simulink model "AcasXuClosedLoop_all_2017b"  (Simulink 9.0)
settings: Solver=VariableStepAuto  StartTime=0.0  StopTime=20  RelTol=1e-3
libraries: neural x14040, simulink x2
(part 15 of 534)

== system AcasXuClosedLoop_all_2017b/networks/Networks_m_3/AcasXu_3_3/Layer 3 ==
  "a{2} " : Inport  PortDimensions=50
      mask Display: e = 0.06;c1 = [120 230 180]/255;c2 = ([120 230 180]+0)/(256+128);c3 = ([120 230 180]+128)/(256+128);patch([0 1 1 0],[0 0 1 1],c1);patch([0 1 1 1-e 1-e 0+e],[0 0 1 1-e 0+e 0+e],c2);patch([0 0 1 1-e 0+e 0+e],[0 1 1 1-e 1-e 0+e],c3);plot([0 1 1 0 0],[0 0 1 1 0]);
  Delays 1 : Subsystem
      mask Display: e = 0.06;c1 = [120 150 230]/255;c2 = ([120 150 230]+0)/383;c3 = ([120 150 230]+128)/383;patch([0 1 1 0],[0 0 1 1],c1);patch([0 1 1 1-e 1-e 0+e],[0 0 1 1-e 0+e 0+e],c2);patch([0 0 1 1-e 0+e 0+e],[0 1 1 1-e 1-e 0+e],c3);plot([0 1 1 0 0],[0 0 1 1 0]);disp('0');
  "LW{3,2}" : Subsystem
      mask Display: e = 0.06;c1 = [120 150 230]/255;c2 = ([120 150 230]+0)/383;c3 = ([120 150 230]+128)/383;patch([0 1 1 0],[0 0 1 1],c1);patch([0 1 1 1-e 1-e 0+e],[0 0 1 1-e 0+e 0+e],c2);patch([0 0 1 1-e 0+e 0+e],[0 1 1 1-e 1-e 0+e],c3);plot([0 1 1 0 0],[0 0 1 1 0]);disp('W');
  "b{3}" : Constant  Value=[1.457030000000000047322146201622672379016876220703125;0.269340999999999997083222069704788736999034881591796875;-0.0234063000000000014100276501949338125996291637420654296875;-1.55862999999999995992538970313034951686859130859375;-0.578284000000000020236257114447653293609619140625;-0.79902899999999998925659383530728518962860107421875;-1.995579999999999909476855464163236320018768310546875;-2.194290000000000073754335971898399293422698974609375;-0.2367740000000000122337695529495249502360820770263671875;-0.71987100000000003863220854327664710581302642822265625;-0.10746999999999999608757406122094835154712200164794921875;-1.548810000000000020037305148434825241565704345703125;-1.0187399999999999788968807479250244796276092529296875;-0.328844999999999998419042412933777086436748504638671875;-1.9179999999999999271693695845897309482097625732421875;-1.38178000000000000824229573481716215610504150390625;-1.721340000000000092228447101661004126071929931640625;-1.3627000000000000223820961764431558549404144287109375;-0.007110700000000000180200299126909158076159656047821044921875;-0.8580069999999999641460135535453446209430694580078125;0.2330230000000000079030115784917143173515796661376953125;0.6196960000000000245989895120146684348583221435546875;0.478331000000000006178169087434071116149425506591796875;-1.4873700000000000809308176030754111707210540771484375;0.499203000000000007840839089112705551087856292724609375;0.1549939999999999928892435718807973898947238922119140625;-0.72241400000000000058975047068088315427303314208984375;-0.9493819999999999481588019989430904388427734375;0.0788308000000000064222405171676655299961566925048828125;0.66645100000000001561062390464940108358860015869140625;0.2498390000000000055191407000165781937539577484130859375;-0.0307758999999999983965270899943789117969572544097900390625;0.60483399999999998275512780310236848890781402587890625;-1.92368000000000005655920176650397479534149169921875;-0.333158000000000009688250202088966034352779388427734375;-0.58032399999999995099386751462589018046855926513671875;-0.422524999999999983923970603427733294665813446044921875;-1.3587100000000000843414227347238920629024505615234375;-0.50581399999999998584598870365880429744720458984375;-1.099110000000000031405988920596428215503692626953125;0.245719999999999993978150314433150924742221832275390625;-1.059979999999999922266624707845039665699005126953125;0.105677999999999994162891425730776973068714141845703125;-0.512558999999999986840748533722944557666778564453125;-0.53886000000000000564881474929279647767543792724609375;0.335861000000000020637713760152109898626804351806640625;-2.210109999999999796926886119763366878032684326171875;1.146900000000000030553337637684307992458343505859375;-2.13619000000000003325340003357268869876861572265625;-1.1020000000000000905941988094127736985683441162109375]
      mask Display: e = 0.06;c1 = [120 150 230]/255;c2 = ([120 150 230]+0)/383;c3 = ([120 150 230]+128)/383;patch([0 1 1 0],[0 0 1 1],c1);patch([0 1 1 1-e 1-e 0+e],[0 0 1 1-e 0+e 0+e],c2);patch([0 0 1 1-e 0+e 0+e],[0 1 1 1-e 1-e 0+e],c3);plot([0 1 1 0 0],[0 0 1 1 0]);disp('b');
  netsum : Sum  Inputs=2  SaturateOnIntegerOverflow=on  InputSameDT=on  OutDataTypeStr=Inherit: Same as first input
      mask Display: e = 0.06;c1 = [120 150 230]/255;c2 = ([120 150 230]+0)/383;c3 = ([120 150 230]+128)/383;patch([0 1 1 0],[0 0 1 1],c1);patch([0 1 1 1-e 1-e 0+e],[0 0 1 1-e 0+e 0+e],c2);patch([0 0 1 1-e 0+e 0+e],[0 1 1 1-e 1-e 0+e],c3);plot([0 1 1 0 0],[0 0 1 1 0]);disp('+');
  poslin : neural/Transfer Functions/poslin
      settings: SourceProductName=Deep Learning Toolbox  SourceProductBaseCode=NN
  "a{3}" : Outport  InitialOutput=[0;0;0;0;0;0;0;0;0;0;0;0;0;0;0;0;0;0;0;0;0;0;0;0;0;0;0;0;0;0;0;0;0;0;0;0;0;0;0;0;0;0;0;0;0;0;0;0;0;0]
      mask Display: e = 0.06;c1 = [120 230 180]/255;c2 = ([120 230 180]+0)/(256+128);c3 = ([120 230 180]+128)/(256+128);patch([0 1 1 0],[0 0 1 1],c1);patch([0 1 1 1-e 1-e 0+e],[0 0 1 1-e 0+e 0+e],c2);patch([0 0 1 1-e 0+e 0+e],[0 1 1 1-e 1-e 0+e],c3);plot([0 1 1 0 0],[0 0 1 1 0]);
  wires:
  poslin/1 -> "a{3}"/1
  netsum/1 -> poslin/1
  "b{3}"/1 -> netsum/2
  "LW{3,2}"/1("lz{3,2}") -> netsum/1
  Delays 1/1("pd{3,2}") -> "LW{3,2}"/1("ad{3,2}")
  "a{2} "/1 -> Delays 1/1("p{3}")

== system AcasXuClosedLoop_all_2017b/networks/Networks_m_3/AcasXu_3_3/Layer 4 ==
  "a{3} " : Inport  PortDimensions=50
      mask Display: e = 0.06;c1 = [120 230 180]/255;c2 = ([120 230 180]+0)/(256+128);c3 = ([120 230 180]+128)/(256+128);patch([0 1 1 0],[0 0 1 1],c1);patch([0 1 1 1-e 1-e 0+e],[0 0 1 1-e 0+e 0+e],c2);patch([0 0 1 1-e 0+e 0+e],[0 1 1 1-e 1-e 0+e],c3);plot([0 1 1 0 0],[0 0 1 1 0]);
  Delays 1 : Subsystem
      mask Display: e = 0.06;c1 = [120 150 230]/255;c2 = ([120 150 230]+0)/383;c3 = ([120 150 230]+128)/383;patch([0 1 1 0],[0 0 1 1],c1);patch([0 1 1 1-e 1-e 0+e],[0 0 1 1-e 0+e 0+e],c2);patch([0 0 1 1-e 0+e 0+e],[0 1 1 1-e 1-e 0+e],c3);plot([0 1 1 0 0],[0 0 1 1 0]);disp('0');
  "LW{4,3}" : Subsystem
      mask Display: e = 0.06;c1 = [120 150 230]/255;c2 = ([120 150 230]+0)/383;c3 = ([120 150 230]+128)/383;patch([0 1 1 0],[0 0 1 1],c1);patch([0 1 1 1-e 1-e 0+e],[0 0 1 1-e 0+e 0+e],c2);patch([0 0 1 1-e 0+e 0+e],[0 1 1 1-e 1-e 0+e],c3);plot([0 1 1 0 0],[0 0 1 1 0]);disp('W');
  "b{4}" : Constant  Value=[0.71493099999999998317434801720082759857177734375;0.02434619999999999839790376654491410590708255767822265625;-1.1054600000000001092104184863273985683917999267578125;0.0755138999999999949164219970043632201850414276123046875;0.1088739999999999985558218895675963722169399261474609375;0.34726800000000002111022467943257652223110198974609375;0.246087000000000000188293824976426549255847930908203125;0.0086718999999999997474464663582693901844322681427001953125;0.94580399999999997806554574708570726215839385986328125;-1.1706499999999999683808482586755417287349700927734375;-0.5646409999999999484998625121079385280609130859375;-0.779117999999999977234210746246390044689178466796875;-0.4107439999999999979962694851565174758434295654296875;0.710671000000000052665427574538625776767730712890625;0.8943090000000000205915284823277033865451812744140625;-0.40828900000000001302424834648263640701770782470703125;-2.18846000000000007190692485892213881015777587890625;1.024010000000000086828322309884242713451385498046875;1.003929999999999989057641869294457137584686279296875;0.403766000000000013780976360067143104970455169677734375;1.5085900000000000975575176198617555201053619384765625;-1.2966599999999999237587644529412500560283660888671875;0.547646999999999994912514011957682669162750244140625;-2.286960000000000103881347968126647174358367919921875;-0.01220700000000000083610895984520539059303700923919677734375;-0.3039169999999999927098315311013720929622650146484375;1.2535600000000000076028072726330719888210296630859375;-0.562553999999999998493649400188587605953216552734375;-0.12156999999999999750865953274114872328937053680419921875;0.69034099999999998242827814465272240340709686279296875;-0.10991299999999999681410400853565079160034656524658203125;0.422111999999999987220888897354598157107830047607421875;-0.457382000000000010775380587801919318735599517822265625;-0.274575999999999986744825264395331032574176788330078125;0.3055309999999999970299313645227812230587005615234375;-1.1061199999999999921129756330628879368305206298828125;0.905712999999999990308197084232233464717864990234375;0.27538099999999998690469737994135357439517974853515625;-0.2375750000000000083932860661661834456026554107666015625;0.79457199999999994499688682481064461171627044677734375;-1.9045900000000000051869619710487313568592071533203125;0.16324600000000000221689333557151257991790771484375;0.11122700000000000641886543917280505411326885223388671875;1.0326299999999999368327507909270934760570526123046875;1.5072700000000001097077984013594686985015869140625;0.32911400000000001764277612892328761518001556396484375;1.3003899999999999348432311307988129556179046630859375;0.25018499999999999072741729833069257438182830810546875;1.8247400000000000286348722511320374906063079833984375;0.362935999999999980847320557586499489843845367431640625]
      mask Display: e = 0.06;c1 = [120 150 230]/255;c2 = ([120 150 230]+0)/383;c3 = ([120 150 230]+128)/383;patch([0 1 1 0],[0 0 1 1],c1);patch([0 1 1 1-e 1-e 0+e],[0 0 1 1-e 0+e 0+e],c2);patch([0 0 1 1-e 0+e 0+e],[0 1 1 1-e 1-e 0+e],c3);plot([0 1 1 0 0],[0 0 1 1 0]);disp('b');
  netsum : Sum  Inputs=2  SaturateOnIntegerOverflow=on  InputSameDT=on  OutDataTypeStr=Inherit: Same as first input
      mask Display: e = 0.06;c1 = [120 150 230]/255;c2 = ([120 150 230]+0)/383;c3 = ([120 150 230]+128)/383;patch([0 1 1 0],[0 0 1 1],c1);patch([0 1 1 1-e 1-e 0+e],[0 0 1 1-e 0+e 0+e],c2);patch([0 0 1 1-e 0+e 0+e],[0 1 1 1-e 1-e 0+e],c3);plot([0 1 1 0 0],[0 0 1 1 0]);disp('+');
  poslin : neural/Transfer Functions/poslin
      settings: SourceProductName=Deep Learning Toolbox  SourceProductBaseCode=NN
  "a{4}" : Outport  InitialOutput=[0;0;0;0;0;0;0;0;0;0;0;0;0;0;0;0;0;0;0;0;0;0;0;0;0;0;0;0;0;0;0;0;0;0;0;0;0;0;0;0;0;0;0;0;0;0;0;0;0;0]
      mask Display: e = 0.06;c1 = [120 230 180]/255;c2 = ([120 230 180]+0)/(256+128);c3 = ([120 230 180]+128)/(256+128);patch([0 1 1 0],[0 0 1 1],c1);patch([0 1 1 1-e 1-e 0+e],[0 0 1 1-e 0+e 0+e],c2);patch([0 0 1 1-e 0+e 0+e],[0 1 1 1-e 1-e 0+e],c3);plot([0 1 1 0 0],[0 0 1 1 0]);
  wires:
  poslin/1 -> "a{4}"/1
  netsum/1 -> poslin/1
  "b{4}"/1 -> netsum/2
  "LW{4,3}"/1("lz{4,3}") -> netsum/1
  Delays 1/1("pd{4,3}") -> "LW{4,3}"/1("ad{4,3}")
  "a{3} "/1 -> Delays 1/1("p{4}")

== system AcasXuClosedLoop_all_2017b/networks/Networks_m_3/AcasXu_3_3/Layer 5 ==
  "a{4} " : Inport  PortDimensions=50
      mask Display: e = 0.06;c1 = [120 230 180]/255;c2 = ([120 230 180]+0)/(256+128);c3 = ([120 230 180]+128)/(256+128);patch([0 1 1 0],[0 0 1 1],c1);patch([0 1 1 1-e 1-e 0+e],[0 0 1 1-e 0+e 0+e],c2);patch([0 0 1 1-e 0+e 0+e],[0 1 1 1-e 1-e 0+e],c3);plot([0 1 1 0 0],[0 0 1 1 0]);
  Delays 1 : Subsystem
      mask Display: e = 0.06;c1 = [120 150 230]/255;c2 = ([120 150 230]+0)/383;c3 = ([120 150 230]+128)/383;patch([0 1 1 0],[0 0 1 1],c1);patch([0 1 1 1-e 1-e 0+e],[0 0 1 1-e 0+e 0+e],c2);patch([0 0 1 1-e 0+e 0+e],[0 1 1 1-e 1-e 0+e],c3);plot([0 1 1 0 0],[0 0 1 1 0]);disp('0');
  "LW{5,4}" : Subsystem
      mask Display: e = 0.06;c1 = [120 150 230]/255;c2 = ([120 150 230]+0)/383;c3 = ([120 150 230]+128)/383;patch([0 1 1 0],[0 0 1 1],c1);patch([0 1 1 1-e 1-e 0+e],[0 0 1 1-e 0+e 0+e],c2);patch([0 0 1 1-e 0+e 0+e],[0 1 1 1-e 1-e 0+e],c3);plot([0 1 1 0 0],[0 0 1 1 0]);disp('W');
  "b{5}" : Constant  Value=[0.1411189999999999944435558063560165464878082275390625;-0.08221900000000000041655567883935873396694660186767578125;0.47773100000000001674749228186556138098239898681640625;1.2366800000000000014921397450962103903293609619140625;0.68673700000000004184386170891229994595050811767578125;-2.747520000000000184314785656169988214969635009765625;-2.62267999999999990023979989928193390369415283203125;-0.44178499999999998326671857284964062273502349853515625;0.50526800000000005042011252953670918941497802734375;0.295632999999999979134912564404658041894435882568359375;0.70687500000000003108624468950438313186168670654296875;1.251349999999999962341235004714690148830413818359375;0.2476069999999999937667638505445211194455623626708984375;-0.032298000000000000320188320301895146258175373077392578125;-0.255309000000000008157030606525950133800506591796875;-0.03110380000000000089155349769498570822179317474365234375;-0.093037800000000003830535888482700102031230926513671875;-0.0255745999999999994167776407039127661846578121185302734375;0.01323709999999999990805132910054453532211482524871826171875;-1.55783000000000004803268893738277256488800048828125;1.490650000000000030553337637684307992458343505859375;1.6610400000000000719779791324981488287448883056640625;0.34136100000000002552269506850279867649078369140625;-0.47167300000000000892441676114685833454132080078125;0.98957700000000003992539632236002944409847259521484375;-0.049506899999999999517630300260861986316740512847900390625;1.60648999999999997356781022972427308559417724609375;0.10922800000000000564259750035489560104906558990478515625;1.590100000000000068922645368729718029499053955078125;-1.5284999999999999698019337301957421004772186279296875;-1.85986999999999991217691786005161702632904052734375;0.37357899999999999440802866956801153719425201416015625;0.48918499999999998095745468162931501865386962890625;1.9168499999999999428013097713119350373744964599609375;1.475379999999999913740111878723837435245513916015625;-0.1451780000000000014903633882568101398646831512451171875;-1.4023399999999999199218336798367090523242950439453125;-0.280540999999999984826359877843060530722141265869140625;1.2049099999999999255351212923415005207061767578125;0.61131400000000002403766075076418928802013397216796875;-1.645399999999999973709918776876293122768402099609375;-0.88296600000000002861355596905923448503017425537109375;0.038886499999999997456701095188691397197544574737548828125;-0.315809999999999979625187052079127170145511627197265625;-1.0611399999999999721467247582040727138519287109375;0.870122999999999979792164594982750713825225830078125;-0.04864000000000000267785793539587757550179958343505859375;0.6608870000000000022311041902867145836353302001953125;-0.27579399999999998360777908601448871195316314697265625;-0.2108919999999999961293184469468542374670505523681640625]
      mask Display: e = 0.06;c1 = [120 150 230]/255;c2 = ([120 150 230]+0)/383;c3 = ([120 150 230]+128)/383;patch([0 1 1 0],[0 0 1 1],c1);patch([0 1 1 1-e 1-e 0+e],[0 0 1 1-e 0+e 0+e],c2);patch([0 0 1 1-e 0+e 0+e],[0 1 1 1-e 1-e 0+e],c3);plot([0 1 1 0 0],[0 0 1 1 0]);disp('b');
  netsum : Sum  Inputs=2  SaturateOnIntegerOverflow=on  InputSameDT=on  OutDataTypeStr=Inherit: Same as first input
      mask Display: e = 0.06;c1 = [120 150 230]/255;c2 = ([120 150 230]+0)/383;c3 = ([120 150 230]+128)/383;patch([0 1 1 0],[0 0 1 1],c1);patch([0 1 1 1-e 1-e 0+e],[0 0 1 1-e 0+e 0+e],c2);patch([0 0 1 1-e 0+e 0+e],[0 1 1 1-e 1-e 0+e],c3);plot([0 1 1 0 0],[0 0 1 1 0]);disp('+');
  poslin : neural/Transfer Functions/poslin
      settings: SourceProductName=Deep Learning Toolbox  SourceProductBaseCode=NN
  "a{5}" : Outport  InitialOutput=[0;0;0;0;0;0;0;0;0;0;0;0;0;0;0;0;0;0;0;0;0;0;0;0;0;0;0;0;0;0;0;0;0;0;0;0;0;0;0;0;0;0;0;0;0;0;0;0;0;0]
      mask Display: e = 0.06;c1 = [120 230 180]/255;c2 = ([120 230 180]+0)/(256+128);c3 = ([120 230 180]+128)/(256+128);patch([0 1 1 0],[0 0 1 1],c1);patch([0 1 1 1-e 1-e 0+e],[0 0 1 1-e 0+e 0+e],c2);patch([0 0 1 1-e 0+e 0+e],[0 1 1 1-e 1-e 0+e],c3);plot([0 1 1 0 0],[0 0 1 1 0]);
  wires:
  poslin/1 -> "a{5}"/1
  netsum/1 -> poslin/1
  "b{5}"/1 -> netsum/2
  "LW{5,4}"/1("lz{5,4}") -> netsum/1
  Delays 1/1("pd{5,4}") -> "LW{5,4}"/1("ad{5,4}")
  "a{4} "/1 -> Delays 1/1("p{5}")

== system AcasXuClosedLoop_all_2017b/networks/Networks_m_3/AcasXu_3_3/Layer 6 ==
  "a{5} " : Inport  PortDimensions=50
      mask Display: e = 0.06;c1 = [120 230 180]/255;c2 = ([120 230 180]+0)/(256+128);c3 = ([120 230 180]+128)/(256+128);patch([0 1 1 0],[0 0 1 1],c1);patch([0 1 1 1-e 1-e 0+e],[0 0 1 1-e 0+e 0+e],c2);patch([0 0 1 1-e 0+e 0+e],[0 1 1 1-e 1-e 0+e],c3);plot([0 1 1 0 0],[0 0 1 1 0]);
  Delays 1 : Subsystem
      mask Display: e = 0.06;c1 = [120 150 230]/255;c2 = ([120 150 230]+0)/383;c3 = ([120 150 230]+128)/383;patch([0 1 1 0],[0 0 1 1],c1);patch([0 1 1 1-e 1-e 0+e],[0 0 1 1-e 0+e 0+e],c2);patch([0 0 1 1-e 0+e 0+e],[0 1 1 1-e 1-e 0+e],c3);plot([0 1 1 0 0],[0 0 1 1 0]);disp('0');
  "LW{6,5}" : Subsystem
      mask Display: e = 0.06;c1 = [120 150 230]/255;c2 = ([120 150 230]+0)/383;c3 = ([120 150 230]+128)/383;patch([0 1 1 0],[0 0 1 1],c1);patch([0 1 1 1-e 1-e 0+e],[0 0 1 1-e 0+e 0+e],c2);patch([0 0 1 1-e 0+e 0+e],[0 1 1 1-e 1-e 0+e],c3);plot([0 1 1 0 0],[0 0 1 1 0]);disp('W');
  "b{6}" : Constant  Value=[0.0584027000000000018342660723646986298263072967529296875;-0.00940746999999999937436268027113328571431338787078857421875;-2.12145999999999990137666827649809420108795166015625;0.050215900000000000813482614603344700299203395843505859375;0.252966999999999997417177155512035824358463287353515625;0.460220000000000017958967646336532197892665863037109375;-0.09918480000000000351700890632855589501559734344482421875;-0.39645399999999997309174659676500596106052398681640625;-0.29279800000000000270716782324598170816898345947265625;0.12137799999999999978061993033406906761229038238525390625;-0.1408409999999999939745976007543504238128662109375;0.0309935999999999996223909448644917574711143970489501953125;-0.53306200000000003580424845495144836604595184326171875;0.201145999999999991469934457199997268617153167724609375;0.1025729999999999975113240680002490989863872528076171875;0.496703999999999978864906324815819971263408660888671875;-0.6736180000000000500648411616566590964794158935546875;-1.3283300000000000107291953099775128066539764404296875;-0.369808999999999998831157199674635194242000579833984375;-0.38796799999999997954347463746671564877033233642578125;0.9658189999999999830748720341944135725498199462890625;-0.9138319999999999776463255329872481524944305419921875;-0.351239000000000023415935856974101625382900238037109375;-0.07563060000000000615560935557368793524801731109619140625;-0.91776800000000002821565203703357838094234466552734375;-0.0708036999999999971944220078512444160878658294677734375;-0.8075229999999999908055769992643035948276519775390625;0.162553000000000003044675622732029296457767486572265625;-0.418551999999999979618081624721526168286800384521484375;-0.0215522999999999999298783137646751129068434238433837890625;-0.0169398000000000013842704760236301808618009090423583984375;-0.448612999999999984002130304361344315111637115478515625;-1.382169999999999898676605880609713494777679443359375;-0.0945635999999999976584064143025898374617099761962890625;-0.032442699999999997928501471733397920615971088409423828125;-1.830130000000000034532376957940869033336639404296875;-0.61505200000000004312283863328048028051853179931640625;0.55395600000000000395772303818375803530216217041015625;-0.1922919999999999907114300867760903201997280120849609375;0.392324999999999979305442820987082086503505706787109375;-0.7704860000000000042064129956997931003570556640625;-0.139174999999999993161026168309035710990428924560546875;0.016413299999999998723954064416830078698694705963134765625;0.2254529999999999867466016212347312830388545989990234375;-0.47548299999999998899369302307604812085628509521484375;0.0243151999999999986201704160748704452998936176300048828125;-0.01274480000000000057269744502264074981212615966796875;0.094808000000000003382183422218076884746551513671875;0.2147199999999999942001949193581822328269481658935546875;0.269589000000000023060664489094051532447338104248046875]
      mask Display: e = 0.06;c1 = [120 150 230]/255;c2 = ([120 150 230]+0)/383;c3 = ([120 150 230]+128)/383;patch([0 1 1 0],[0 0 1 1],c1);patch([0 1 1 1-e 1-e 0+e],[0 0 1 1-e 0+e 0+e],c2);patch([0 0 1 1-e 0+e 0+e],[0 1 1 1-e 1-e 0+e],c3);plot([0 1 1 0 0],[0 0 1 1 0]);disp('b');
  netsum : Sum  Inputs=2  SaturateOnIntegerOverflow=on  InputSameDT=on  OutDataTypeStr=Inherit: Same as first input
      mask Display: e = 0.06;c1 = [120 150 230]/255;c2 = ([120 150 230]+0)/383;c3 = ([120 150 230]+128)/383;patch([0 1 1 0],[0 0 1 1],c1);patch([0 1 1 1-e 1-e 0+e],[0 0 1 1-e 0+e 0+e],c2);patch([0 0 1 1-e 0+e 0+e],[0 1 1 1-e 1-e 0+e],c3);plot([0 1 1 0 0],[0 0 1 1 0]);disp('+');
  poslin : neural/Transfer Functions/poslin
      settings: SourceProductName=Deep Learning Toolbox  SourceProductBaseCode=NN
  "a{6}" : Outport  InitialOutput=[0;0;0;0;0;0;0;0;0;0;0;0;0;0;0;0;0;0;0;0;0;0;0;0;0;0;0;0;0;0;0;0;0;0;0;0;0;0;0;0;0;0;0;0;0;0;0;0;0;0]
      mask Display: e = 0.06;c1 = [120 230 180]/255;c2 = ([120 230 180]+0)/(256+128);c3 = ([120 230 180]+128)/(256+128);patch([0 1 1 0],[0 0 1 1],c1);patch([0 1 1 1-e 1-e 0+e],[0 0 1 1-e 0+e 0+e],c2);patch([0 0 1 1-e 0+e 0+e],[0 1 1 1-e 1-e 0+e],c3);plot([0 1 1 0 0],[0 0 1 1 0]);
  wires:
  poslin/1 -> "a{6}"/1
  netsum/1 -> poslin/1
  "b{6}"/1 -> netsum/2
  "LW{6,5}"/1("lz{6,5}") -> netsum/1
  Delays 1/1("pd{6,5}") -> "LW{6,5}"/1("ad{6,5}")
  "a{5} "/1 -> Delays 1/1("p{6}")

== system AcasXuClosedLoop_all_2017b/networks/Networks_m_3/AcasXu_3_3/Layer 7 ==
  "a{6} " : Inport  PortDimensions=50
      mask Display: e = 0.06;c1 = [120 230 180]/255;c2 = ([120 230 180]+0)/(256+128);c3 = ([120 230 180]+128)/(256+128);patch([0 1 1 0],[0 0 1 1],c1);patch([0 1 1 1-e 1-e 0+e],[0 0 1 1-e 0+e 0+e],c2);patch([0 0 1 1-e 0+e 0+e],[0 1 1 1-e 1-e 0+e],c3);plot([0 1 1 0 0],[0 0 1 1 0]);
  Delays 1 : Subsystem
      mask Display: e = 0.06;c1 = [120 150 230]/255;c2 = ([120 150 230]+0)/383;c3 = ([120 150 230]+128)/383;patch([0 1 1 0],[0 0 1 1],c1);patch([0 1 1 1-e 1-e 0+e],[0 0 1 1-e 0+e 0+e],c2);patch([0 0 1 1-e 0+e 0+e],[0 1 1 1-e 1-e 0+e],c3);plot([0 1 1 0 0],[0 0 1 1 0]);disp('0');
  "LW{7,6}" : Subsystem
      mask Display: e = 0.06;c1 = [120 150 230]/255;c2 = ([120 150 230]+0)/383;c3 = ([120 150 230]+128)/383;patch([0 1 1 0],[0 0 1 1],c1);patch([0 1 1 1-e 1-e 0+e],[0 0 1 1-e 0+e 0+e],c2);patch([0 0 1 1-e 0+e 0+e],[0 1 1 1-e 1-e 0+e],c3);plot([0 1 1 0 0],[0 0 1 1 0]);disp('W');
  "b{7}" : Constant  Value=[0.01963979999999999892512647647890844382345676422119140625;0.0208072000000000013886225502801607945002615451812744140625;-0.0233902999999999992863930486919343820773065090179443359375;0.020034900000000001318856135412715957500040531158447265625;-0.0164280000000000016291412663349547074176371097564697265625]
      mask Display: e = 0.06;c1 = [120 150 230]/255;c2 = ([120 150 230]+0)/383;c3 = ([120 150 230]+128)/383;patch([0 1 1 0],[0 0 1 1],c1);patch([0 1 1 1-e 1-e 0+e],[0 0 1 1-e 0+e 0+e],c2);patch([0 0 1 1-e 0+e 0+e],[0 1 1 1-e 1-e 0+e],c3);plot([0 1 1 0 0],[0 0 1 1 0]);disp('b');
  netsum : Sum  Inputs=2  SaturateOnIntegerOverflow=on  InputSameDT=on  OutDataTypeStr=Inherit: Same as first input
      mask Display: e = 0.06;c1 = [120 150 230]/255;c2 = ([120 150 230]+0)/383;c3 = ([120 150 230]+128)/383;patch([0 1 1 0],[0 0 1 1],c1);patch([0 1 1 1-e 1-e 0+e],[0 0 1 1-e 0+e 0+e],c2);patch([0 0 1 1-e 0+e 0+e],[0 1 1 1-e 1-e 0+e],c3);plot([0 1 1 0 0],[0 0 1 1 0]);disp('+');
  purelin : neural/Transfer Functions/purelin
      settings: SourceProductName=Deep Learning Toolbox
  "a{7}" : Outport  InitialOutput=[0;0;0;0;0]
      mask Display: e = 0.06;c1 = [120 230 180]/255;c2 = ([120 230 180]+0)/(256+128);c3 = ([120 230 180]+128)/(256+128);patch([0 1 1 0],[0 0 1 1],c1);patch([0 1 1 1-e 1-e 0+e],[0 0 1 1-e 0+e 0+e],c2);patch([0 0 1 1-e 0+e 0+e],[0 1 1 1-e 1-e 0+e],c3);plot([0 1 1 0 0],[0 0 1 1 0]);
  wires:
  purelin/1 -> "a{7}"/1
  netsum/1 -> purelin/1
  "b{7}"/1 -> netsum/2
  "LW{7,6}"/1("lz{7,6}") -> netsum/1
  Delays 1/1("pd{7,6}") -> "LW{7,6}"/1("ad{7,6}")
  "a{6} "/1 -> Delays 1/1("p{7}")

== system AcasXuClosedLoop_all_2017b/networks/Networks_m_3/AcasXu_3_3/Process Input 1 ==
  x : Inport  PortDimensions=5
      mask Display: e = 0.06;c1 = [120 230 180]/255;c2 = ([120 230 180]+0)/(256+128);c3 = ([120 230 180]+128)/(256+128);patch([0 1 1 0],[0 0 1 1],c1);patch([0 1 1 1-e 1-e 0+e],[0 0 1 1-e 0+e 0+e],c2);patch([0 0 1 1-e 0+e 0+e],[0 1 1 1-e 1-e 0+e],c3);plot([0 1 1 0 0],[0 0 1 1 0]);
  p : Outport  PortDimensions=5
      mask Display: e = 0.06;c1 = [120 230 180]/255;c2 = ([120 230 180]+0)/(256+128);c3 = ([120 230 180]+128)/(256+128);patch([0 1 1 0],[0 0 1 1],c1);patch([0 1 1 1-e 1-e 0+e],[0 0 1 1-e 0+e 0+e],c2);patch([0 0 1 1-e 0+e 0+e],[0 1 1 1-e 1-e 0+e],c3);plot([0 1 1 0 0],[0 0 1 1 0]);
  wires:
  x/1 -> p/1

== system AcasXuClosedLoop_all_2017b/networks/Networks_m_3/AcasXu_3_3/Process Output 1 ==
  a : Inport  PortDimensions=5
      mask Display: e = 0.06;c1 = [120 230 180]/255;c2 = ([120 230 180]+0)/(256+128);c3 = ([120 230 180]+128)/(256+128);patch([0 1 1 0],[0 0 1 1],c1);patch([0 1 1 1-e 1-e 0+e],[0 0 1 1-e 0+e 0+e],c2);patch([0 0 1 1-e 0+e 0+e],[0 1 1 1-e 1-e 0+e],c3);plot([0 1 1 0 0],[0 0 1 1 0]);
  y : Outport  PortDimensions=5
      mask Display: e = 0.06;c1 = [120 230 180]/255;c2 = ([120 230 180]+0)/(256+128);c3 = ([120 230 180]+128)/(256+128);patch([0 1 1 0],[0 0 1 1],c1);patch([0 1 1 1-e 1-e 0+e],[0 0 1 1-e 0+e 0+e],c2);patch([0 0 1 1-e 0+e 0+e],[0 1 1 1-e 1-e 0+e],c3);plot([0 1 1 0 0],[0 0 1 1 0]);
  wires:
  a/1 -> y/1

== system AcasXuClosedLoop_all_2017b/networks/Networks_m_3/AcasXu_4_3/Layer 1 ==
  "p{1}" : Inport  PortDimensions=5
      mask Display: e = 0.06;c1 = [120 230 180]/255;c2 = ([120 230 180]+0)/(256+128);c3 = ([120 230 180]+128)/(256+128);patch([0 1 1 0],[0 0 1 1],c1);patch([0 1 1 1-e 1-e 0+e],[0 0 1 1-e 0+e 0+e],c2);patch([0 0 1 1-e 0+e 0+e],[0 1 1 1-e 1-e 0+e],c3);plot([0 1 1 0 0],[0 0 1 1 0]);
  Delays 1 : Subsystem
      mask Display: e = 0.06;c1 = [120 150 230]/255;c2 = ([120 150 230]+0)/383;c3 = ([120 150 230]+128)/383;patch([0 1 1 0],[0 0 1 1],c1);patch([0 1 1 1-e 1-e 0+e],[0 0 1 1-e 0+e 0+e],c2);patch([0 0 1 1-e 0+e 0+e],[0 1 1 1-e 1-e 0+e],c3);plot([0 1 1 0 0],[0 0 1 1 0]);disp('0');
  "IW{1,1}" : Subsystem
      mask Display: e = 0.06;c1 = [120 150 230]/255;c2 = ([120 150 230]+0)/383;c3 = ([120 150 230]+128)/383;patch([0 1 1 0],[0 0 1 1],c1);patch([0 1 1 1-e 1-e 0+e],[0 0 1 1-e 0+e 0+e],c2);patch([0 0 1 1-e 0+e 0+e],[0 1 1 1-e 1-e 0+e],c3);plot([0 1 1 0 0],[0 0 1 1 0]);disp('W');
  "b{1}" : Constant  Value=[-0.3111990000000000033963942769332788884639739990234375;0.273482000000000002870592652470804750919342041015625;0.490464999999999984314769108095788396894931793212890625;0.105013999999999996237676214150269515812397003173828125;-0.476953000000000015834444866413832642138004302978515625;0.322998999999999980570208890640060417354106903076171875;0.2372599999999999986766141546468134038150310516357421875;0.20890100000000000335376171278767287731170654296875;0.036893500000000002680966559864828013814985752105712890625;-0.85636699999999998933475353624089621007442474365234375;0.051341900000000002812772237348326598294079303741455078125;-0.1213930000000000009041656312547274865210056304931640625;-0.3881419999999999870254896450205706059932708740234375;-0.01363959999999999998798738687355580623261630535125732421875;-0.10042399999999999937738692779021221213042736053466796875;-0.431796999999999986386001182836480438709259033203125;-0.24927799999999999958077978590154089033603668212890625;-0.13099199999999999732835931354202330112457275390625;-0.40817300000000000803623834144673310220241546630859375;-0.2413980000000000014637180356658063828945159912109375;-0.306088999999999999968025576890795491635799407958984375;0.0980229999999999990212273814904619939625263214111328125;0.047538900000000001988542663866610382683575153350830078125;0.254294999999999993267607578673050738871097564697265625;-0.55544099999999996253308154336991719901561737060546875;-0.28488400000000002609823468446847982704639434814453125;0.1164300000000000057109872386718052439391613006591796875;0.26525900000000002254552100566797889769077301025390625;-0.1455490000000000117008625011294498108327388763427734375;-0.69714299999999995716137846102355979382991790771484375;0.1464560000000000028475000135586014948785305023193359375;0.03360559999999999936104444486772990785539150238037109375;0.025910099999999998410249446578745846636593341827392578125;-0.734064000000000049794834922067821025848388671875;-0.171792000000000000259348098552436567842960357666015625;0.0340190999999999965641706012320355512201786041259765625;-0.62583800000000000540723021913436241447925567626953125;-0.1301170000000000104289910041188704781234264373779296875;-0.46083499999999999463540234501124359667301177978515625;-0.2914820000000000188578042070730589330196380615234375;0.30190099999999997493205228238366544246673583984375;-0.52053599999999999869970679355901665985584259033203125;-0.471853000000000022406965172194759361445903778076171875;0.338031000000000025895730004776851274073123931884765625;-0.724307000000000034134473025915212929248809814453125;0.11774300000000000043787196091216173954308032989501953125;-0.220098999999999989096721719761262647807598114013671875;0.0215890999999999999570121644865139387547969818115234375;-0.07961010000000000308428838025065488182008266448974609375;-0.2167469999999999952233764588527265004813671112060546875]
      mask Display: e = 0.06;c1 = [120 150 230]/255;c2 = ([120 150 230]+0)/383;c3 = ([120 150 230]+128)/383;patch([0 1 1 0],[0 0 1 1],c1);patch([0 1 1 1-e 1-e 0+e],[0 0 1 1-e 0+e 0+e],c2);patch([0 0 1 1-e 0+e 0+e],[0 1 1 1-e 1-e 0+e],c3);plot([0 1 1 0 0],[0 0 1 1 0]);disp('b');
  netsum : Sum  Inputs=2  SaturateOnIntegerOverflow=on  InputSameDT=on  OutDataTypeStr=Inherit: Same as first input
      mask Display: e = 0.06;c1 = [120 150 230]/255;c2 = ([120 150 230]+0)/383;c3 = ([120 150 230]+128)/383;patch([0 1 1 0],[0 0 1 1],c1);patch([0 1 1 1-e 1-e 0+e],[0 0 1 1-e 0+e 0+e],c2);patch([0 0 1 1-e 0+e 0+e],[0 1 1 1-e 1-e 0+e],c3);plot([0 1 1 0 0],[0 0 1 1 0]);disp('+');
  poslin : neural/Transfer Functions/poslin
      settings: SourceProductName=Deep Learning Toolbox  SourceProductBaseCode=NN
  "a{1}" : Outport  InitialOutput=[0;0;0;0;0;0;0;0;0;0;0;0;0;0;0;0;0;0;0;0;0;0;0;0;0;0;0;0;0;0;0;0;0;0;0;0;0;0;0;0;0;0;0;0;0;0;0;0;0;0]
      mask Display: e = 0.06;c1 = [120 230 180]/255;c2 = ([120 230 180]+0)/(256+128);c3 = ([120 230 180]+128)/(256+128);patch([0 1 1 0],[0 0 1 1],c1);patch([0 1 1 1-e 1-e 0+e],[0 0 1 1-e 0+e 0+e],c2);patch([0 0 1 1-e 0+e 0+e],[0 1 1 1-e 1-e 0+e],c3);plot([0 1 1 0 0],[0 0 1 1 0]);
  wires:
  poslin/1 -> "a{1}"/1
  netsum/1 -> poslin/1
  "b{1}"/1 -> netsum/2
  "IW{1,1}"/1("iz{1,1}") -> netsum/1
  Delays 1/1("pd{1,1}") -> "IW{1,1}"/1("pd{1,1}")
  "p{1}"/1 -> Delays 1/1("p{1}")

== system AcasXuClosedLoop_all_2017b/networks/Networks_m_3/AcasXu_4_3/Layer 2 ==
  "a{1} " : Inport  PortDimensions=50
      mask Display: e = 0.06;c1 = [120 230 180]/255;c2 = ([120 230 180]+0)/(256+128);c3 = ([120 230 180]+128)/(256+128);patch([0 1 1 0],[0 0 1 1],c1);patch([0 1 1 1-e 1-e 0+e],[0 0 1 1-e 0+e 0+e],c2);patch([0 0 1 1-e 0+e 0+e],[0 1 1 1-e 1-e 0+e],c3);plot([0 1 1 0 0],[0 0 1 1 0]);
  Delays 1 : Subsystem
      mask Display: e = 0.06;c1 = [120 150 230]/255;c2 = ([120 150 230]+0)/383;c3 = ([120 150 230]+128)/383;patch([0 1 1 0],[0 0 1 1],c1);patch([0 1 1 1-e 1-e 0+e],[0 0 1 1-e 0+e 0+e],c2);patch([0 0 1 1-e 0+e 0+e],[0 1 1 1-e 1-e 0+e],c3);plot([0 1 1 0 0],[0 0 1 1 0]);disp('0');
  "LW{2,1}" : Subsystem
      mask Display: e = 0.06;c1 = [120 150 230]/255;c2 = ([120 150 230]+0)/383;c3 = ([120 150 230]+128)/383;patch([0 1 1 0],[0 0 1 1],c1);patch([0 1 1 1-e 1-e 0+e],[0 0 1 1-e 0+e 0+e],c2);patch([0 0 1 1-e 0+e 0+e],[0 1 1 1-e 1-e 0+e],c3);plot([0 1 1 0 0],[0 0 1 1 0]);disp('W');
  "b{2}" : Constant  Value=[-0.32989400000000002055600134553969837725162506103515625;-0.404455999999999982197351755530689842998981475830078125;-0.0232659999999999984876541958556117606349289417266845703125;-0.74153199999999996894217701992602087557315826416015625;-0.379442000000000001502797886132611893117427825927734375;0.2495610000000000050501824944149120710790157318115234375;-0.059748900000000000620747897528417524881660938262939453125;1.3816800000000000192557081390987150371074676513671875;1.7353799999999999226218960757250897586345672607421875;-0.29258499999999998397726130860974080860614776611328125;-0.57733699999999998908606357872486114501953125;-0.1979349999999999998312461002569762058556079864501953125;-0.0804736000000000062382099486057995818555355072021484375;-1.102019999999999999573674358543939888477325439453125;-0.007517099999999999650202031631351928808726370334625244140625;-0.1924269999999999869455535872475593350827693939208984375;-0.04406580000000000219007034729656879790127277374267578125;-1.170139999999999957935870043002068996429443359375;0.1255469999999999919371163059622631408274173736572265625;-0.487810000000000021369572777985013090074062347412109375;-0.285144000000000008565592679588007740676403045654296875;-0.010062200000000000310595993369133793748915195465087890625;0.15712599999999998789945721000549383461475372314453125;-1.1877299999999999524646909776492975652217864990234375;0.50656000000000001026734253173344768583774566650390625;0.54265600000000002722799763432703912258148193359375;-0.7177670000000000438689085058285854756832122802734375;-0.01499660000000000055486726324716073577292263507843017578125;0.850832000000000032713387554395012557506561279296875;1.3803199999999999914024328973027877509593963623046875;-0.54020199999999995998933854934875853359699249267578125;0.50198299999999995701926991387153975665569305419921875;-0.414658000000000026563640176391345448791980743408203125;-0.393753999999999992898125356077798642218112945556640625;0.59867400000000003945643811675836332142353057861328125;0.402388000000000023437252139046904630959033966064453125;-1.0117000000000000436983782492461614310741424560546875;0.07722389999999999810054163162931217812001705169677734375;-0.368508999999999975472064761561341583728790283203125;-0.1843889999999999973478992387754260562360286712646484375;-0.01012739999999999994384491941445958218537271022796630859375;-1.0836900000000000421351842305739410221576690673828125;-1.2413600000000000189714910447946749627590179443359375;0.6315880000000000382698317480389960110187530517578125;-0.0173430000000000007764899834228344843722879886627197265625;-0.64253499999999996727950701824738644063472747802734375;-0.06925589999999999524238347703430918045341968536376953125;0.31874599999999997379518390516750514507293701171875;-0.132805000000000006377121053446899168193340301513671875;-0.0248102000000000010027090269204563810490071773529052734375]
      mask Display: e = 0.06;c1 = [120 150 230]/255;c2 = ([120 150 230]+0)/383;c3 = ([120 150 230]+128)/383;patch([0 1 1 0],[0 0 1 1],c1);patch([0 1 1 1-e 1-e 0+e],[0 0 1 1-e 0+e 0+e],c2);patch([0 0 1 1-e 0+e 0+e],[0 1 1 1-e 1-e 0+e],c3);plot([0 1 1 0 0],[0 0 1 1 0]);disp('b');
  netsum : Sum  Inputs=2  SaturateOnIntegerOverflow=on  InputSameDT=on  OutDataTypeStr=Inherit: Same as first input
      mask Display: e = 0.06;c1 = [120 150 230]/255;c2 = ([120 150 230]+0)/383;c3 = ([120 150 230]+128)/383;patch([0 1 1 0],[0 0 1 1],c1);patch([0 1 1 1-e 1-e 0+e],[0 0 1 1-e 0+e 0+e],c2);patch([0 0 1 1-e 0+e 0+e],[0 1 1 1-e 1-e 0+e],c3);plot([0 1 1 0 0],[0 0 1 1 0]);disp('+');
  poslin : neural/Transfer Functions/poslin
      settings: SourceProductName=Deep Learning Toolbox  SourceProductBaseCode=NN
  "a{2}" : Outport  InitialOutput=[0;0;0;0;0;0;0;0;0;0;0;0;0;0;0;0;0;0;0;0;0;0;0;0;0;0;0;0;0;0;0;0;0;0;0;0;0;0;0;0;0;0;0;0;0;0;0;0;0;0]
      mask Display: e = 0.06;c1 = [120 230 180]/255;c2 = ([120 230 180]+0)/(256+128);c3 = ([120 230 180]+128)/(256+128);patch([0 1 1 0],[0 0 1 1],c1);patch([0 1 1 1-e 1-e 0+e],[0 0 1 1-e 0+e 0+e],c2);patch([0 0 1 1-e 0+e 0+e],[0 1 1 1-e 1-e 0+e],c3);plot([0 1 1 0 0],[0 0 1 1 0]);
  wires:
  poslin/1 -> "a{2}"/1
  netsum/1 -> poslin/1
  "b{2}"/1 -> netsum/2
  "LW{2,1}"/1("lz{2,1}") -> netsum/1
  Delays 1/1("pd{2,1}") -> "LW{2,1}"/1("ad{2,1}")
  "a{1} "/1 -> Delays 1/1("p{2}")

== system AcasXuClosedLoop_all_2017b/networks/Networks_m_3/AcasXu_4_3/Layer 3 ==
  "a{2} " : Inport  PortDimensions=50
      mask Display: e = 0.06;c1 = [120 230 180]/255;c2 = ([120 230 180]+0)/(256+128);c3 = ([120 230 180]+128)/(256+128);patch([0 1 1 0],[0 0 1 1],c1);patch([0 1 1 1-e 1-e 0+e],[0 0 1 1-e 0+e 0+e],c2);patch([0 0 1 1-e 0+e 0+e],[0 1 1 1-e 1-e 0+e],c3);plot([0 1 1 0 0],[0 0 1 1 0]);
  Delays 1 : Subsystem
      mask Display: e = 0.06;c1 = [120 150 230]/255;c2 = ([120 150 230]+0)/383;c3 = ([120 150 230]+128)/383;patch([0 1 1 0],[0 0 1 1],c1);patch([0 1 1 1-e 1-e 0+e],[0 0 1 1-e 0+e 0+e],c2);patch([0 0 1 1-e 0+e 0+e],[0 1 1 1-e 1-e 0+e],c3);plot([0 1 1 0 0],[0 0 1 1 0]);disp('0');
  "LW{3,2}" : Subsystem
      mask Display: e = 0.06;c1 = [120 150 230]/255;c2 = ([120 150 230]+0)/383;c3 = ([120 150 230]+128)/383;patch([0 1 1 0],[0 0 1 1],c1);patch([0 1 1 1-e 1-e 0+e],[0 0 1 1-e 0+e 0+e],c2);patch([0 0 1 1-e 0+e 0+e],[0 1 1 1-e 1-e 0+e],c3);plot([0 1 1 0 0],[0 0 1 1 0]);disp('W');
  "b{3}" : Constant  Value=[-1.9056599999999999095479097377392463386058807373046875;-0.315439000000000024925839170464314520359039306640625;-1.0443400000000000460431692772544920444488525390625;-1.4550099999999999145217088880599476397037506103515625;0.25647100000000000452615722679183818399906158447265625;-0.1478170000000000039452885403079562820494174957275390625;0.3674589999999999800905925440019927918910980224609375;-0.1504620000000000124007470958531484939157962799072265625;0.800729000000000024073187887552194297313690185546875;0.51093200000000005278621983961784280836582183837890625;-0.72134299999999995645083572526345960795879364013671875;-0.373699999999999976640907561886706389486789703369140625;-0.34145599999999998175326254568062722682952880859375;-0.8357900000000000328981286656926386058330535888671875;-0.357385000000000008224532166423159651458263397216796875;-0.96121500000000004160227717875386588275432586669921875;0.096247799999999994469135344843380153179168701171875;-0.88066400000000000236610731008113361895084381103515625;-0.77947200000000005370992539610597304999828338623046875;-0.412949999999999983746334919487708248198032379150390625;-1.204730000000000078586026575067080557346343994140625;0.060769600000000000006306066779870889149606227874755859375;-0.286856999999999973116615592516609467566013336181640625;-0.88954699999999997661603856613510288298130035400390625;0.40067799999999997861976908097858540713787078857421875;1.4197699999999999764810354463406838476657867431640625;-0.30375099999999999322852772820624522864818572998046875;-0.407828999999999997072563928668387234210968017578125;-0.845995999999999970242470226367004215717315673828125;-0.77757299999999995865351820611977018415927886962890625;-0.25088100000000002065547732854611240327358245849609375;-0.81788600000000000189714910447946749627590179443359375;-0.787576000000000053802295951754786074161529541015625;1.0043599999999999194955080383806489408016204833984375;-2.20746999999999982122744768275879323482513427734375;0.1855070000000000052242654646761366166174411773681640625;-0.65790800000000004832401145904441364109516143798828125;-0.6767250000000000209610107049229554831981658935546875;0.35265000000000001900701818158267997205257415771484375;0.268382999999999982687626243205158971250057220458984375;0.50165000000000004032330025438568554818630218505859375;-0.3782360000000000166409108715015463531017303466796875;-0.46989999999999998436805981327779591083526611328125;-1.7535199999999999675992512493394315242767333984375;-0.292148000000000018783197219818248413503170013427734375;-0.26247100000000000985522774499258957803249359130859375;-1.80177999999999993718802215880714356899261474609375;0.3497689999999999965751840136363171041011810302734375;-0.48502699999999998592414840459241531789302825927734375;-1.231640000000000068069994085817597806453704833984375]
      mask Display: e = 0.06;c1 = [120 150 230]/255;c2 = ([120 150 230]+0)/383;c3 = ([120 150 230]+128)/383;patch([0 1 1 0],[0 0 1 1],c1);patch([0 1 1 1-e 1-e 0+e],[0 0 1 1-e 0+e 0+e],c2);patch([0 0 1 1-e 0+e 0+e],[0 1 1 1-e 1-e 0+e],c3);plot([0 1 1 0 0],[0 0 1 1 0]);disp('b');
  netsum : Sum  Inputs=2  SaturateOnIntegerOverflow=on  InputSameDT=on  OutDataTypeStr=Inherit: Same as first input
      mask Display: e = 0.06;c1 = [120 150 230]/255;c2 = ([120 150 230]+0)/383;c3 = ([120 150 230]+128)/383;patch([0 1 1 0],[0 0 1 1],c1);patch([0 1 1 1-e 1-e 0+e],[0 0 1 1-e 0+e 0+e],c2);patch([0 0 1 1-e 0+e 0+e],[0 1 1 1-e 1-e 0+e],c3);plot([0 1 1 0 0],[0 0 1 1 0]);disp('+');
  poslin : neural/Transfer Functions/poslin
      settings: SourceProductName=Deep Learning Toolbox  SourceProductBaseCode=NN
  "a{3}" : Outport  InitialOutput=[0;0;0;0;0;0;0;0;0;0;0;0;0;0;0;0;0;0;0;0;0;0;0;0;0;0;0;0;0;0;0;0;0;0;0;0;0;0;0;0;0;0;0;0;0;0;0;0;0;0]
      mask Display: e = 0.06;c1 = [120 230 180]/255;c2 = ([120 230 180]+0)/(256+128);c3 = ([120 230 180]+128)/(256+128);patch([0 1 1 0],[0 0 1 1],c1);patch([0 1 1 1-e 1-e 0+e],[0 0 1 1-e 0+e 0+e],c2);patch([0 0 1 1-e 0+e 0+e],[0 1 1 1-e 1-e 0+e],c3);plot([0 1 1 0 0],[0 0 1 1 0]);
  wires:
  poslin/1 -> "a{3}"/1
  netsum/1 -> poslin/1
  "b{3}"/1 -> netsum/2
  "LW{3,2}"/1("lz{3,2}") -> netsum/1
  Delays 1/1("pd{3,2}") -> "LW{3,2}"/1("ad{3,2}")
  "a{2} "/1 -> Delays 1/1("p{3}")

== system AcasXuClosedLoop_all_2017b/networks/Networks_m_3/AcasXu_4_3/Layer 4 ==
  "a{3} " : Inport  PortDimensions=50
      mask Display: e = 0.06;c1 = [120 230 180]/255;c2 = ([120 230 180]+0)/(256+128);c3 = ([120 230 180]+128)/(256+128);patch([0 1 1 0],[0 0 1 1],c1);patch([0 1 1 1-e 1-e 0+e],[0 0 1 1-e 0+e 0+e],c2);patch([0 0 1 1-e 0+e 0+e],[0 1 1 1-e 1-e 0+e],c3);plot([0 1 1 0 0],[0 0 1 1 0]);
  Delays 1 : Subsystem
      mask Display: e = 0.06;c1 = [120 150 230]/255;c2 = ([120 150 230]+0)/383;c3 = ([120 150 230]+128)/383;patch([0 1 1 0],[0 0 1 1],c1);patch([0 1 1 1-e 1-e 0+e],[0 0 1 1-e 0+e 0+e],c2);patch([0 0 1 1-e 0+e 0+e],[0 1 1 1-e 1-e 0+e],c3);plot([0 1 1 0 0],[0 0 1 1 0]);disp('0');
  "LW{4,3}" : Subsystem
      mask Display: e = 0.06;c1 = [120 150 230]/255;c2 = ([120 150 230]+0)/383;c3 = ([120 150 230]+128)/383;patch([0 1 1 0],[0 0 1 1],c1);patch([0 1 1 1-e 1-e 0+e],[0 0 1 1-e 0+e 0+e],c2);patch([0 0 1 1-e 0+e 0+e],[0 1 1 1-e 1-e 0+e],c3);plot([0 1 1 0 0],[0 0 1 1 0]);disp('W');
  "b{4}" : Constant  Value=[-0.0228053999999999999770405878507517627440392971038818359375;0.544076000000000004064304448547773063182830810546875;1.028259999999999951825202515465207397937774658203125;-0.43240000000000000657252030578092671930789947509765625;-1.2398700000000000276401124210678972303867340087890625;0.51781200000000005001510317015345208346843719482421875;-2.960669999999999912887460595811717212200164794921875;-0.8408189999999999830748720341944135725498199462890625;-0.5612500000000000266453525910037569701671600341796875;-2.403420000000000111839426608639769256114959716796875;0.0207474999999999985822451975536750978790223598480224609375;-1.1033699999999999619149093632586300373077392578125;0.809541999999999983828047334100119769573211669921875;-0.74963400000000002254552100566797889769077301025390625;-0.11881899999999999406607997798346332274377346038818359375;-1.3342300000000000270716782324598170816898345947265625;0.1424020000000000008011369345695129595696926116943359375;-1.9183600000000000651567688692011870443820953369140625;-0.34782999999999997253752326287212781608104705810546875;0.289351999999999998092192754484131000936031341552734375;-1.4103300000000000835598257253877818584442138671875;0.5560709999999999819664253664086572825908660888671875;-2.28742000000000000881072992342524230480194091796875;1.1674100000000000587618842473602853715419769287109375;0.1539870000000000127560184637331985868513584136962890625;0.7444509999999999738662381787435151636600494384765625;-0.77071699999999998542676848956034518778324127197265625;-0.444313000000000013489653838405502028763294219970703125;-1.109609999999999985220711096189916133880615234375;0.03750040000000000317559312179582775570452213287353515625;0.42820300000000000029132252166164107620716094970703125;-1.424840000000000106439301816863007843494415283203125;0.09503810000000000035580427493187016807496547698974609375;1.052890000000000103597130873822607100009918212890625;0.6713329999999999575521769656916148960590362548828125;-0.93754700000000001924860271174111403524875640869140625;0.72352300000000002722089220696943812072277069091796875;1.420230000000000103455022326670587062835693359375;2.009399999999999852917653697659261524677276611328125;-0.7310520000000000351292328559793531894683837890625;0.55549700000000001853805997598101384937763214111328125;-1.3734699999999999686650653529795818030834197998046875;0.144409000000000009578116078046150505542755126953125;-0.110648999999999997356781022972427308559417724609375;-0.783668000000000031235458664014004170894622802734375;1.14932999999999996276756064617075026035308837890625;-4.96309000000000022367885321727953851222991943359375;-0.1513750000000000095479180117763462476432323455810546875;-0.40402199999999999224797875285730697214603424072265625;-0.01779669999999999863149469092604704201221466064453125]
      mask Display: e = 0.06;c1 = [120 150 230]/255;c2 = ([120 150 230]+0)/383;c3 = ([120 150 230]+128)/383;patch([0 1 1 0],[0 0 1 1],c1);patch([0 1 1 1-e 1-e 0+e],[0 0 1 1-e 0+e 0+e],c2);patch([0 0 1 1-e 0+e 0+e],[0 1 1 1-e 1-e 0+e],c3);plot([0 1 1 0 0],[0 0 1 1 0]);disp('b');
  netsum : Sum  Inputs=2  SaturateOnIntegerOverflow=on  InputSameDT=on  OutDataTypeStr=Inherit: Same as first input
      mask Display: e = 0.06;c1 = [120 150 230]/255;c2 = ([120 150 230]+0)/383;c3 = ([120 150 230]+128)/383;patch([0 1 1 0],[0 0 1 1],c1);patch([0 1 1 1-e 1-e 0+e],[0 0 1 1-e 0+e 0+e],c2);patch([0 0 1 1-e 0+e 0+e],[0 1 1 1-e 1-e 0+e],c3);plot([0 1 1 0 0],[0 0 1 1 0]);disp('+');
  poslin : neural/Transfer Functions/poslin
      settings: SourceProductName=Deep Learning Toolbox  SourceProductBaseCode=NN
  "a{4}" : Outport  InitialOutput=[0;0;0;0;0;0;0;0;0;0;0;0;0;0;0;0;0;0;0;0;0;0;0;0;0;0;0;0;0;0;0;0;0;0;0;0;0;0;0;0;0;0;0;0;0;0;0;0;0;0]
      mask Display: e = 0.06;c1 = [120 230 180]/255;c2 = ([120 230 180]+0)/(256+128);c3 = ([120 230 180]+128)/(256+128);patch([0 1 1 0],[0 0 1 1],c1);patch([0 1 1 1-e 1-e 0+e],[0 0 1 1-e 0+e 0+e],c2);patch([0 0 1 1-e 0+e 0+e],[0 1 1 1-e 1-e 0+e],c3);plot([0 1 1 0 0],[0 0 1 1 0]);
  wires:
  poslin/1 -> "a{4}"/1
  netsum/1 -> poslin/1
  "b{4}"/1 -> netsum/2
  "LW{4,3}"/1("lz{4,3}") -> netsum/1
  Delays 1/1("pd{4,3}") -> "LW{4,3}"/1("ad{4,3}")
  "a{3} "/1 -> Delays 1/1("p{4}")
